simulink model "Final_V2"  (R2024a)
settings: Solver=FixedStepAuto  StartTime=0.0  StopTime=inf  FixedStep=1e-3  RelTol=1e-3
libraries: fl_lib x107, nesl_utility x75, ee_lib x16, batteryecm_lib x14, simulink x2
(part 16 of 16)

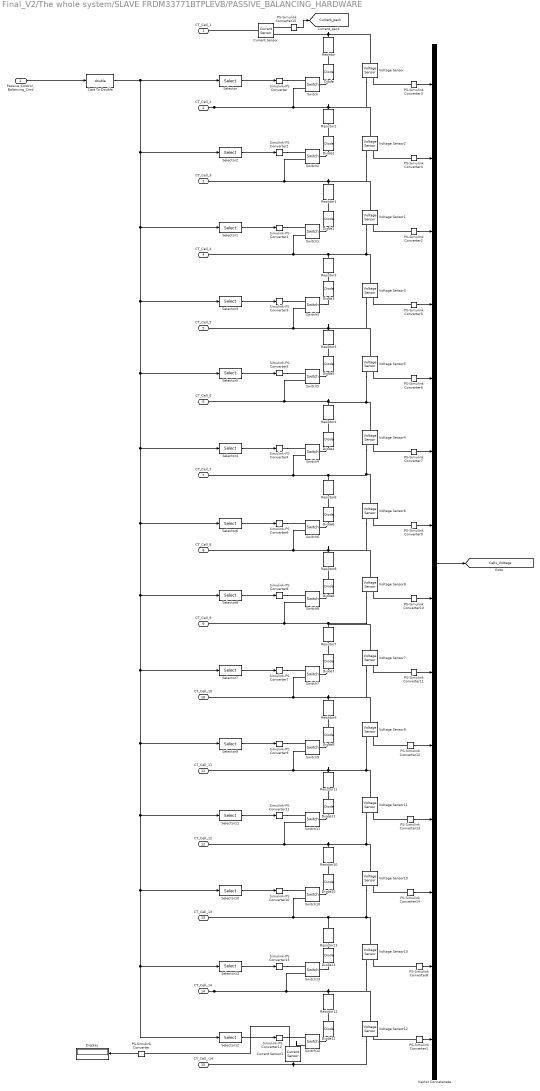
== system Final_V2/The whole system/SLAVE FRDM33771BTPLEVB/PASSIVE_BALANCING_HARDWARE ==
  Passive_Control_Balancing_Cmd : Inport
  Cast To Double : DataTypeConversion  OutDataTypeStr=double  SaturateOnIntegerOverflow=off  RndMeth=Floor
  Current Sensor : fl_lib/Electrical/Electrical Sensors/Current Sensor
  Current Sensor1 : fl_lib/Electrical/Electrical Sensors/Current Sensor
  Diode{,1..13} (14 alike) : ee_lib/Semiconductors & Converters/Diode  ModelType=ee.enum.diode.modelType.pwl  TableType=ee.enum.diode.tableType.IfMat  IfMat=[.07, .12, .19, 1.75, 4.24, 7.32, 11.2; .16, .3, .72, 2.14, 5.02, 8.35, 13.12] A  VfMat=[.9, 1.15, 1.25, 1.5, 1.75, 2.17, 2.6, 2.85; .58, .68, .75, 1.1, 1.38, 1.77, 2.27, 2.7] V  TjVec=[25, 125] degC  VfVec=[.5, .7, .9, 1.3, 1.7, 2.1, 2.5] V  IfVec=[.1, .2, .5, 1, 2, 4, 7, 10] A  IrMat=[1e-06, 2e-06, 5e-06, 1e-05, 2.5e-05, 5e-05, .0001; 2e-06, 5e-06, 1e-05, 4e-05, 8e-05, .0002, .0005] A  VrMat=[10, 100, 200, 250, 300, 325, 350; 10, 70, 160, 220, 270, 300, 320] V  VrVec=[10, 20, 40, 50, 70, 80, 100] V  IrVec=[1e-06, 2e-06, 5e-06, 1e-05, 2e-05, 5e-05, .0001] A  Vf=0.6 V  Ron=0.3 Ohm  Goff=1e-8 S  prmExp=ee.enum.diode.expParam.useTwo  I12=[.0137, .545] A  V12=[.6, .7] V  RS=0 Ohm  Tmeas=298.15 K  IS=1e-12 A  ec=1  I1=0.0137 A  V1=0.6 V  N_series=1  N_parallel=1  ZenerEnable=false  Rz=0.3 Ohm  BV=inf V  C_param=ee.enum.diode.capParam.fixed  CJ=0 pF  CJ0=5 pF  Vvec=[.1, 10, 100] V  Cvec=[3.5, 1, .4] pF  VJ=1 V  Mgrad=0.5  FC=0.5  Q_param=ee.enum.diode.recoveryParam.off  iRM=-7.15 A  iF=4 A  didt=-750 A/us  trr_factor=3  trr=115 ns  Qrr=150 nA*s  Vr=-600 V  Ls=150 nH  Erec=0.03 J  TT=50 ns  tau=100 ns  T_param=ee.enum.diode.temperatureParam.off  Tdevice=298.15 K  I1_T2=0.245 A  V1_T2=0.5 V  IS_T2=1.25e-7 A  Tmeas2=125 degC  XTI_param=ee.enum.diode.xtiParam.pn  XTI=3  EG_param=ee.enum.diode.egapParam.material_si  EG=1.11 eV  dBVdT=0 V/K  enable_faults=simscape.enum.onoff.off  fault_action=simscape.enum.assert.action.none  fault_types=ee.enum.diode.faults.open  tau_fault=1e-3 s  IfMat_faulted=[.07, .12, .19, 1.75, 4.24, 7.32, 11.2; .16, .3, .72, 2.14, 4.02, 6.35, 9.12] A  VfMat_faulted=[.9, 1.15, 1.25, 1.5, 1.75, 2.17, 2.6, 2.85; .58, .68, .75, 1.1, 1.38, 1.77, 2.27, 2.7] V  VfVec_faulted=[.5, .7, .9, 1.3, 1.7, 2.1, 2.5] V  IfVec_faulted=[.1, .2, .5, 1, 2, 4, 7, 10] A  IrMat_faulted=[1e-06, 2e-06, 5e-06, 1e-05, 2.5e-05, 5e-05, .0001; 2e-06, 5e-06, 1e-05, 4e-05, 8e-05, .0002, .0005] A  VrMat_faulted=[1, 10, 20, 25, 30, 32.5, 35; 1, 5, 10, 20, 25, 30, 32] V  VrVec_faulted=[1, 2, 4, 5, 7, 8, 10] V  IrVec_faulted=[1e-06, 2e-06, 5e-06, 1e-05, 2e-05, 5e-05, .0001] A  Ropen_faulted=1000 Ohm  Gshort_faulted=1000 1/Ohm  Vf_faulted=0.6 V  Ron_faulted=0.3 Ohm  Goff_faulted=1e-8 S  I12_faulted=[.0137, .545] A  V12_faulted=[.6, .7] V  IS_faulted=1e-12 A  ec_faulted=1  I1_faulted=0.0137 A  V1_faulted=0.6 V  RS_faulted=0 Ohm  Rz_faulted=0.3 Ohm  BV_faulted=inf V  Cscale_faulted=100  fault_trigger=ee.enum.faults.faultTrigger.temporal  t_fault=inf s  max_permissible_voltage=inf V  n_fail_on_voltage=1  max_permissible_current=inf A  t_fail_on_current=1 s  max_permissible_dVdt=inf V/s  enable_fault_input=simscape.enum.onoff.off  i_specify=off  i_priority=None  i=0 A  i_nominal_specify=off  i_nominal_value=1  i_nominal_unit=A  v_specify=off  v_priority=None  v=0 V  v_nominal_specify=off  v_nominal_value=1  v_nominal_unit=V  v_capacitor_specify=off  v_capacitor_priority=None  v_capacitor=0 V  v_capacitor_nominal_specify=off  v_capacitor_nominal_value=1  v_capacitor_nominal_unit=V  Qj_specify=off  Qj_priority=High  Qj=0 C  Qj_nominal_specify=off  Qj_nominal_value=1  Qj_nominal_unit=C  faulted_specify=off  faulted_priority=None  faulted=0  faulted_nominal_specify=off  faulted_nominal_value=1  faulted_nominal_unit=1  i_diode_specify=off  i_diode_priority=None  i_diode=0 A  i_diode_nominal_specify=off  i_diode_nominal_value=1  i_diode_nominal_unit=A  v_diode_specify=off  v_diode_priority=None  v_diode=0 V  v_diode_nominal_specify=off  v_diode_nominal_value=1  v_diode_nominal_unit=V  i_capacitor_specify=off  i_capacitor_priority=None  i_capacitor=0 A  i_capacitor_nominal_specify=off  i_capacitor_nominal_value=1  i_capacitor_nominal_unit=A  power_dissipated_specify=off  power_dissipated_priority=None  power_dissipated=0 W  power_dissipated_nominal_specify=off  power_dissipated_nominal_value=1  power_dissipated_nominal_unit=W  fidelity_level=ee.enum.diode.fidelity.includeChargeDyn  rec_loss_model=ee.enum.diode.reverseRecoveryModel.fixed  ErecVal=0 J  scaleWithIandV=true  ErecMat=zeros(2, 3) J  TjrecVec=[298.15, 398.15] K  IfrecVec=[.1, 1, 10] A  IrecVal=10 A  VrecVal=10 V  tFilter=1 / 1000 / 100 s  max_permissible_temperature=inf K  t_fail_on_temperature=1 s  thermal_network_parameterization=ee.enum.thermalNetworkTopology.junctionCase  Rth=[0, 10] K/W  Rth_Cauer=[1, 3, 10] K/W  Rth_Foster=[4, 6] K/W  thermal_mass_parameterization=ee.enum.thermalNetwork.timeconstant  thermal_mass=[0, 1] J/K  thermal_mass_Cauer=[.1, .3, 1] J/K  thermal_mass_Foster=[1.5, 3] J/K  thermal_time_constant=[0, 10] s  thermal_time_constant_Cauer=[1, 3, 10] s  thermal_time_constant_Foster=[6, 18] s  thermal_mass_initial_temperatures=[25, 25] degC  thermal_mass_initial_temperatures_Cauer=[25, 25, 25] degC  junction_thermal_mass=0.01 J/K  Tvec_initial_Foster=[25, 25, 25, 25] degC  no_thermal_network=0  junction_temperature_specify=off  junction_temperature_priority=None  junction_temperature=25 degC  junction_temperature_nominal_specify=off  junction_temperature_nominal_value=1  junction_temperature_nominal_unit=degC
  Display : Display
  PS-Simulink Converter : nesl_utility/PS-Simulink Converter  PhysicalDomain=network_engine_domain  SubClassName=ps_output  LeftPortType=input  RightPortType=output  PseudoPeriodic=off  Frequencies=[]  VectorFormat=inherit  Unit=inherit  AffineConversion=off
  PS-Simulink Converter{1..15} (15 alike) : nesl_utility/PS-Simulink Converter  PhysicalDomain=network_engine_domain  SubClassName=ps_output  LeftPortType=input  RightPortType=output  PseudoPeriodic=off  Frequencies=[]  VectorFormat=1-D array  Unit=inherit  AffineConversion=off
  Resistor{,1..13} (14 alike) : fl_lib/Electrical/Electrical Elements/Resistor  R=1 Ohm  i_specify=off  i_priority=None  i=0 A  i_nominal_specify=off  i_nominal_value=1  i_nominal_unit=A  v_specify=off  v_priority=None  v=0 V  v_nominal_specify=off  v_nominal_value=1  v_nominal_unit=V
  Selector : Selector  InputPortWidth=14  IndexOptions=Index vector (dialog)  Indices=1  OutputSizes=1
  Selector1 : Selector  InputPortWidth=14  IndexOptions=Index vector (dialog)  Indices=3  OutputSizes=1
  Selector10 : Selector  InputPortWidth=14  IndexOptions=Index vector (dialog)  Indices=12  OutputSizes=1
  Selector11 : Selector  InputPortWidth=14  IndexOptions=Index vector (dialog)  Indices=11  OutputSizes=1
  Selector12 : Selector  InputPortWidth=14  IndexOptions=Index vector (dialog)  Indices=14  OutputSizes=1
  Selector13 : Selector  InputPortWidth=14  IndexOptions=Index vector (dialog)  Indices=13  OutputSizes=1
  Selector2 : Selector  InputPortWidth=14  IndexOptions=Index vector (dialog)  Indices=2  OutputSizes=1
  Selector3 : Selector  InputPortWidth=14  IndexOptions=Index vector (dialog)  Indices=4  OutputSizes=1
  Selector4 : Selector  InputPortWidth=14  IndexOptions=Index vector (dialog)  Indices=6  OutputSizes=1
  Selector5 : Selector  InputPortWidth=14  IndexOptions=Index vector (dialog)  Indices=5  OutputSizes=1
  Selector6 : Selector  InputPortWidth=14  IndexOptions=Index vector (dialog)  Indices=7  OutputSizes=1
  Selector7 : Selector  InputPortWidth=14  IndexOptions=Index vector (dialog)  Indices=9  OutputSizes=1
  Selector8 : Selector  InputPortWidth=14  IndexOptions=Index vector (dialog)  Indices=8  OutputSizes=1
  Selector9 : Selector  InputPortWidth=14  IndexOptions=Index vector (dialog)  Indices=10  OutputSizes=1
  Simulink-PS Converter{,1..13} (14 alike) : nesl_utility/Simulink-PS Converter  PhysicalDomain=network_engine_domain  SubClassName=ps_input  LeftPortType=input  RightPortType=output  PseudoPeriodic=off  Frequencies=[]  NoiseDistribution=none  NoiseParameters=[]  Unit=1  AffineConversion=off  FilteringAndDerivatives=provide  SimscapeFilterOrder=1  InputFilterTimeConstant=0.001  UdotUserProvided=0
  Switch{,1..13} (14 alike) : fl_lib/Electrical/Electrical Elements/Switch  R_closed=0.01 Ohm  G_open=1e-8 1/Ohm  Threshold=0.5  i_specify=off  i_priority=None  i=0 A  i_nominal_specify=off  i_nominal_value=1  i_nominal_unit=A  v_specify=off  v_priority=None  v=0 V  v_nominal_specify=off  v_nominal_value=1  v_nominal_unit=V
  Vector Concatenate : Concatenate  NumInputs=14
  Voltage Sensor{,1..13} (14 alike) : fl_lib/Electrical/Electrical Sensors/Voltage Sensor
  CT_Cell_1 : PMIOPort  Side=Left
  CT_Cell_2 : PMIOPort  Port=2  Side=Left
  CT_Cell_3 : PMIOPort  Port=3  Side=Left
  CT_Cell_4 : PMIOPort  Port=4  Side=Left
  CT_Cell_5 : PMIOPort  Port=5  Side=Left
  CT_Cell_6 : PMIOPort  Port=6  Side=Left
  CT_Cell_7 : PMIOPort  Port=7  Side=Left
  CT_Cell_8 : PMIOPort  Port=8  Side=Left
  CT_Cell_9 : PMIOPort  Port=9  Side=Left
  CT_Cell_10 : PMIOPort  Port=10  Side=Left
  CT_Cell_11 : PMIOPort  Port=11  Side=Left
  CT_Cell_12 : PMIOPort  Port=12  Side=Left
  CT_Cell_13 : PMIOPort  Port=13  Side=Left
  CT_Cell_14 : PMIOPort  Port=14  Side=Left
  CT_Cell_-14 : PMIOPort  Port=15  Side=Left
  tag scope: Cells_Voltage=global, Current_pack=global
  wires:
  PS-Simulink Converter11/1 -> Vector Concatenate/9
  PS-Simulink Converter1/1 -> Vector Concatenate/14
  PS-Simulink Converter3/1 -> Vector Concatenate/1
  PS-Simulink Converter14/1 -> Vector Concatenate/12
  PS-Simulink Converter5/1 -> Vector Concatenate/4
  Selector9/1 -> Simulink-PS Converter9/1
  Selector6/1 -> Simulink-PS Converter6/1
  Selector7/1 -> Simulink-PS Converter7/1
  Selector12/1 -> Simulink-PS Converter12/1
  Selector13/1 -> Simulink-PS Converter13/1
  PS-Simulink Converter7/1 -> Vector Concatenate/6
  Selector1/1 -> Simulink-PS Converter1/1
  PS-Simulink Converter9/1 -> Vector Concatenate/7
  PS-Simulink Converter2/1 -> Vector Concatenate/3
  Selector5/1 -> Simulink-PS Converter5/1
  PS-Simulink Converter4/1 -> Vector Concatenate/2
  Selector2/1 -> Simulink-PS Converter2/1
  PS-Simulink Converter10/1 -> Vector Concatenate/8
  Vector Concatenate/1 -> [Cells_Voltage]
  PS-Simulink Converter8/1 -> Vector Concatenate/13
  Selector/1 -> Simulink-PS Converter/1
  PS-Simulink Converter12/1 -> Vector Concatenate/10
  Selector10/1 -> Simulink-PS Converter10/1
  Selector8/1 -> Simulink-PS Converter8/1
  Selector3/1 -> Simulink-PS Converter3/1
  Selector11/1 -> Simulink-PS Converter11/1
  PS-Simulink Converter6/1 -> Vector Concatenate/5
  Selector4/1 -> Simulink-PS Converter4/1
  PS-Simulink Converter13/1 -> Vector Concatenate/11
  Cast To Double/1 -> Selector2/1, Selector1/1, Selector3/1, Selector5/1, Selector4/1, Selector6/1, Selector8/1, Selector9/1, Selector11/1, Selector10/1, Selector13/1, Selector12/1, Selector7/1, Selector/1
  Passive_Control_Balancing_Cmd/1 -> Cast To Double/1
  PS-Simulink Converter15/1 -> [Current_pack]
  PS-Simulink Converter/1 -> Display/1
  connections:
  Voltage Sensor9/RConn2 -- Voltage Sensor11/LConn1 -- Resistor11/LConn1 -- Switch9/RConn2 -- CT_Cell_11/RConn1
  CT_Cell_14/RConn1 -- Switch13/RConn2 -- Voltage Sensor12/LConn1 -- Voltage Sensor13/RConn2 -- Resistor12/LConn1
  Voltage Sensor4/RConn2 -- Voltage Sensor6/LConn1 -- Resistor6/LConn1 -- Switch4/RConn2 -- CT_Cell_7/RConn1
  Resistor1/LConn1 -- Voltage Sensor1/LConn1 -- Voltage Sensor2/RConn2 -- Switch2/RConn2 -- CT_Cell_3/RConn1
  Voltage Sensor4/LConn1 -- Voltage Sensor5/RConn2 -- Resistor4/LConn1 -- Switch5/RConn2 -- CT_Cell_6/RConn1
  Resistor8/LConn1 -- Voltage Sensor8/LConn1 -- Voltage Sensor6/RConn2 -- Switch6/RConn2 -- CT_Cell_8/RConn1
  Switch3/RConn2 -- Voltage Sensor5/LConn1 -- Voltage Sensor3/RConn2 -- Resistor5/LConn1 -- CT_Cell_5/RConn1
  Voltage Sensor11/RConn2 -- Voltage Sensor10/LConn1 -- Resistor10/LConn1 -- Switch11/RConn2 -- CT_Cell_12/RConn1
  Resistor7/LConn1 -- Voltage Sensor8/RConn2 -- Voltage Sensor7/LConn1 -- Switch8/RConn2 -- CT_Cell_9/RConn1
  Voltage Sensor10/RConn2 -- Voltage Sensor13/LConn1 -- Resistor13/LConn1 -- Switch10/RConn2 -- CT_Cell_13/RConn1
  Voltage Sensor12/RConn2 -- Current Sensor1/LConn1 -- CT_Cell_-14/RConn1
  Voltage Sensor1/RConn2 -- Voltage Sensor3/LConn1 -- Resistor3/LConn1 -- Switch1/RConn2 -- CT_Cell_4/RConn1
  Resistor/LConn1 -- Voltage Sensor/LConn1 -- Current Sensor/RConn2
  Resistor9/LConn1 -- Voltage Sensor9/LConn1 -- Voltage Sensor7/RConn2 -- Switch7/RConn2 -- CT_Cell_10/RConn1
  CT_Cell_2/RConn1 -- Voltage Sensor2/LConn1 -- Voltage Sensor/RConn2 -- Resistor2/LConn1 -- Switch/RConn2
  PS-Simulink Converter13/LConn1 -- Voltage Sensor11/RConn1
  Simulink-PS Converter13/RConn1 -- Switch13/RConn1
  Simulink-PS Converter5/RConn1 -- Switch5/RConn1
  PS-Simulink Converter3/LConn1 -- Voltage Sensor/RConn1
  Diode6/LConn1 -- Resistor6/RConn1
  Switch6/LConn1 -- Diode6/RConn1
  PS-Simulink Converter6/LConn1 -- Voltage Sensor5/RConn1
  Diode7/LConn1 -- Resistor7/RConn1
  Diode2/LConn1 -- Resistor2/RConn1
  Diode1/LConn1 -- Resistor1/RConn1
  Switch9/LConn1 -- Diode9/RConn1
  Simulink-PS Converter10/RConn1 -- Switch10/RConn1
  Diode11/LConn1 -- Resistor11/RConn1
  Resistor13/RConn1 -- Diode13/LConn1
  PS-Simulink Converter4/LConn1 -- Voltage Sensor2/RConn1
  Switch10/LConn1 -- Diode10/RConn1
  Switch13/LConn1 -- Diode13/RConn1
  Simulink-PS Converter11/RConn1 -- Switch11/RConn1
  PS-Simulink Converter8/LConn1 -- Voltage Sensor13/RConn1
  Diode9/LConn1 -- Resistor9/RConn1
  Diode3/LConn1 -- Resistor3/RConn1
  Switch3/LConn1 -- Diode3/RConn1
  Diode12/LConn1 -- Resistor12/RConn1
  Simulink-PS Converter2/RConn1 -- Switch2/RConn1
  Simulink-PS Converter6/RConn1 -- Switch6/RConn1
  Simulink-PS Converter1/RConn1 -- Switch1/RConn1
  Switch2/LConn1 -- Diode2/RConn1
  PS-Simulink Converter2/LConn1 -- Voltage Sensor1/RConn1
  Switch5/LConn1 -- Diode5/RConn1
  Switch7/LConn1 -- Diode7/RConn1
  Diode5/LConn1 -- Resistor5/RConn1
  Simulink-PS Converter8/RConn1 -- Switch8/RConn1
  PS-Simulink Converter11/LConn1 -- Voltage Sensor7/RConn1
  Switch/LConn1 -- Diode/RConn1
  Simulink-PS Converter/RConn1 -- Switch/RConn1
  Simulink-PS Converter9/RConn1 -- Switch9/RConn1
  PS-Simulink Converter5/LConn1 -- Voltage Sensor3/RConn1
  PS-Simulink Converter7/LConn1 -- Voltage Sensor4/RConn1
  Diode8/LConn1 -- Resistor8/RConn1
  PS-Simulink Converter10/LConn1 -- Voltage Sensor8/RConn1
  PS-Simulink Converter9/LConn1 -- Voltage Sensor6/RConn1
  Simulink-PS Converter12/RConn1 -- Switch12/RConn1
  PS-Simulink Converter14/LConn1 -- Voltage Sensor10/RConn1
  Switch11/LConn1 -- Diode11/RConn1
  Diode10/LConn1 -- Resistor10/RConn1
  Simulink-PS Converter3/RConn1 -- Switch3/RConn1
  PS-Simulink Converter12/LConn1 -- Voltage Sensor9/RConn1
  Diode4/LConn1 -- Resistor4/RConn1
  Diode/LConn1 -- Resistor/RConn1
  Switch1/LConn1 -- Diode1/RConn1
  Switch12/LConn1 -- Diode12/RConn1
  PS-Simulink Converter1/LConn1 -- Voltage Sensor12/RConn1
  Simulink-PS Converter7/RConn1 -- Switch7/RConn1
  Simulink-PS Converter4/RConn1 -- Switch4/RConn1
  Switch8/LConn1 -- Diode8/RConn1
  Switch4/LConn1 -- Diode4/RConn1
  Current Sensor/LConn1 -- CT_Cell_1/RConn1
  PS-Simulink Converter15/LConn1 -- Current Sensor/RConn1
  Current Sensor1/RConn2 -- Switch12/RConn2
  Current Sensor1/RConn1 -- PS-Simulink Converter/LConn1

== system Final_V2/The whole system/SLAVE FRDM33771BTPLEVB/Tempreture_Of_Cells ==
  Temp.13&14 : Inport
  Temp.11&12 : Inport  Port=2
  Temp.9&10 : Inport  Port=3
  Temp.7&8 : Inport  Port=4
  Temp.5&6 : Inport  Port=5
  Temp.3&4 : Inport  Port=6
  Temp.1&2 : Inport  Port=7
  Bus Creator : BusCreator  Inputs=7
  tag scope: Temp_Sensing=global
  wires:
  Temp.1&2/1 -> Bus Creator/1  "Temp_1&2"
  Temp.3&4/1 -> Bus Creator/2  "Temp_3&4"
  Temp.5&6/1 -> Bus Creator/3  "Temp_5&6"
  Temp.7&8/1 -> Bus Creator/4  "Temp_7&8"
  Temp.9&10/1 -> Bus Creator/5  "Temp_9&10"
  Temp.11&12/1 -> Bus Creator/6  "Temp_11&12"
  Temp.13&14/1 -> Bus Creator/7  "Temp_13&14"
  Bus Creator/1 -> [Temp_Sensing]

== system Final_V2/The whole system/Master/Subsystem15/Subsystem1 ==
  soc_intial_cell_2 : Inport
  Current_Branch : Inport  Port=2
  "\"Ah\"" : Gain  Gain=1/1.5
  Constant : Constant  Value=0
  Integrator : Integrator
  Sum : Sum
  Switch : Switch  Criteria=u2 > Threshold  SaturateOnIntegerOverflow=off  InputSameDT=off
  To convert Ah -->Coulomb : Gain  Gain=1/3600
  SOC_CELL_2 : Outport
  wires:
  soc_intial_cell_2/1 -> Sum/1
  Current_Branch/1 -> Integrator/1
  Integrator/1 -> "\"Ah\""/1  "Integrated current"
  Constant/1 -> Switch/3
  To convert Ah -->Coulomb/1 -> Sum/2  "Integrated current / Coulomb"
  Switch/1 -> SOC_CELL_2/1
  "\"Ah\""/1 -> To convert Ah -->Coulomb/1
  Sum/1 -> Switch/1, Switch/2

== system Final_V2/The whole system/Master/Subsystem15/Subsystem10 ==
  soc_intial_cell_11 : Inport
  Current_Branch : Inport  Port=2
  "\"Ah\"" : Gain  Gain=1/1.5
  Constant : Constant  Value=0
  Integrator : Integrator
  Sum : Sum
  Switch : Switch  Criteria=u2 > Threshold  SaturateOnIntegerOverflow=off  InputSameDT=off
  To convert Ah -->Coulomb : Gain  Gain=1/3600
  SOC_CELL_11 : Outport
  wires:
  Sum/1 -> Switch/2, Switch/1
  "\"Ah\""/1 -> To convert Ah -->Coulomb/1
  Switch/1 -> SOC_CELL_11/1
  To convert Ah -->Coulomb/1 -> Sum/2  "Integrated current / Coulomb"
  Constant/1 -> Switch/3
  Integrator/1 -> "\"Ah\""/1  "Integrated current"
  Current_Branch/1 -> Integrator/1
  soc_intial_cell_11/1 -> Sum/1

== system Final_V2/The whole system/Master/Subsystem15/Subsystem11 ==
  soc_intial_cell_12 : Inport
  Current_Branch : Inport  Port=2
  "\"Ah\"" : Gain  Gain=1/1.5
  Constant : Constant  Value=0
  Integrator : Integrator
  Sum : Sum
  Switch : Switch  Criteria=u2 > Threshold  SaturateOnIntegerOverflow=off  InputSameDT=off
  To convert Ah -->Coulomb : Gain  Gain=1/3600
  SOC_CELL_12 : Outport
  wires:
  soc_intial_cell_12/1 -> Sum/1
  Current_Branch/1 -> Integrator/1
  Integrator/1 -> "\"Ah\""/1  "Integrated current"
  Constant/1 -> Switch/3
  To convert Ah -->Coulomb/1 -> Sum/2  "Integrated current / Coulomb"
  Switch/1 -> SOC_CELL_12/1
  "\"Ah\""/1 -> To convert Ah -->Coulomb/1
  Sum/1 -> Switch/1, Switch/2

== system Final_V2/The whole system/Master/Subsystem15/Subsystem12 ==
  soc_intial_cell_13 : Inport
  Current_Branch : Inport  Port=2
  "\"Ah\"" : Gain  Gain=1/1.5
  Constant : Constant  Value=0
  Integrator : Integrator
  Sum : Sum
  Switch : Switch  Criteria=u2 > Threshold  SaturateOnIntegerOverflow=off  InputSameDT=off
  To convert Ah -->Coulomb : Gain  Gain=1/3600
  SOC_CELL_13 : Outport
  wires:
  Sum/1 -> Switch/2, Switch/1
  "\"Ah\""/1 -> To convert Ah -->Coulomb/1
  Switch/1 -> SOC_CELL_13/1
  To convert Ah -->Coulomb/1 -> Sum/2  "Integrated current / Coulomb"
  Constant/1 -> Switch/3
  Integrator/1 -> "\"Ah\""/1  "Integrated current"
  Current_Branch/1 -> Integrator/1
  soc_intial_cell_13/1 -> Sum/1

== system Final_V2/The whole system/Master/Subsystem15/Subsystem13 ==
  soc_intial_cell_12 : Inport
  Current_Branch : Inport  Port=2
  "\"Ah\"" : Gain  Gain=1/1.5
  Constant : Constant  Value=0
  Integrator : Integrator
  Sum : Sum
  Switch : Switch  Criteria=u2 > Threshold  SaturateOnIntegerOverflow=off  InputSameDT=off
  To convert Ah -->Coulomb : Gain  Gain=1/3600
  SOC_CELL_14 : Outport
  wires:
  soc_intial_cell_12/1 -> Sum/1
  Current_Branch/1 -> Integrator/1
  Integrator/1 -> "\"Ah\""/1  "Integrated current"
  Constant/1 -> Switch/3
  To convert Ah -->Coulomb/1 -> Sum/2  "Integrated current / Coulomb"
  Switch/1 -> SOC_CELL_14/1
  "\"Ah\""/1 -> To convert Ah -->Coulomb/1
  Sum/1 -> Switch/1, Switch/2

== system Final_V2/The whole system/Master/Subsystem15/Subsystem14 ==
  soc_intial_cell_1 : Inport
  Current_Branch : Inport  Port=2
  "\"Ah\"" : Gain  Gain=1/1.5
  Constant : Constant  Value=0
  Integrator : Integrator
  Sum : Sum
  Switch : Switch  Criteria=u2 > Threshold  SaturateOnIntegerOverflow=off  InputSameDT=off
  To convert Ah -->Coulomb : Gain  Gain=1/3600
  SOC_CELL_1 : Outport
  wires:
  Sum/1 -> Switch/2, Switch/1
  "\"Ah\""/1 -> To convert Ah -->Coulomb/1
  Switch/1 -> SOC_CELL_1/1
  To convert Ah -->Coulomb/1 -> Sum/2  "Integrated current / Coulomb"
  Constant/1 -> Switch/3
  Integrator/1 -> "\"Ah\""/1  "Integrated current"
  Current_Branch/1 -> Integrator/1
  soc_intial_cell_1/1 -> Sum/1

== system Final_V2/The whole system/Master/Subsystem15/Subsystem2 ==
  soc_intial_cell_3 : Inport
  Current_Branch : Inport  Port=2
  "\"Ah\"" : Gain  Gain=1/1.5
  Constant : Constant  Value=0
  Integrator : Integrator
  Sum : Sum
  Switch : Switch  Criteria=u2 > Threshold  SaturateOnIntegerOverflow=off  InputSameDT=off
  To convert Ah -->Coulomb : Gain  Gain=1/3600
  SOC_CELL_3 : Outport
  wires:
  Sum/1 -> Switch/2, Switch/1
  "\"Ah\""/1 -> To convert Ah -->Coulomb/1
  Switch/1 -> SOC_CELL_3/1
  To convert Ah -->Coulomb/1 -> Sum/2  "Integrated current / Coulomb"
  Constant/1 -> Switch/3
  Integrator/1 -> "\"Ah\""/1  "Integrated current"
  Current_Branch/1 -> Integrator/1
  soc_intial_cell_3/1 -> Sum/1

== system Final_V2/The whole system/Master/Subsystem15/Subsystem3 ==
  soc_intial_cell_4 : Inport
  Current_Branch : Inport  Port=2
  "\"Ah\"" : Gain  Gain=1/1.5
  Constant : Constant  Value=0
  Integrator : Integrator
  Sum : Sum
  Switch : Switch  Criteria=u2 > Threshold  SaturateOnIntegerOverflow=off  InputSameDT=off
  To convert Ah -->Coulomb : Gain  Gain=1/3600
  SOC_CELL_4 : Outport
  wires:
  soc_intial_cell_4/1 -> Sum/1
  Current_Branch/1 -> Integrator/1
  Integrator/1 -> "\"Ah\""/1  "Integrated current"
  Constant/1 -> Switch/3
  To convert Ah -->Coulomb/1 -> Sum/2  "Integrated current / Coulomb"
  Switch/1 -> SOC_CELL_4/1
  "\"Ah\""/1 -> To convert Ah -->Coulomb/1
  Sum/1 -> Switch/1, Switch/2

== system Final_V2/The whole system/Master/Subsystem15/Subsystem4 ==
  soc_intial_cell_5 : Inport
  Current_Branch : Inport  Port=2
  "\"Ah\"" : Gain  Gain=1/1.5
  Constant : Constant  Value=0
  Integrator : Integrator
  Sum : Sum
  Switch : Switch  Criteria=u2 > Threshold  SaturateOnIntegerOverflow=off  InputSameDT=off
  To convert Ah -->Coulomb : Gain  Gain=1/3600
  SOC_CELL_5 : Outport
  wires:
  Sum/1 -> Switch/2, Switch/1
  "\"Ah\""/1 -> To convert Ah -->Coulomb/1
  Switch/1 -> SOC_CELL_5/1
  To convert Ah -->Coulomb/1 -> Sum/2  "Integrated current / Coulomb"
  Constant/1 -> Switch/3
  Integrator/1 -> "\"Ah\""/1  "Integrated current"
  Current_Branch/1 -> Integrator/1
  soc_intial_cell_5/1 -> Sum/1

== system Final_V2/The whole system/Master/Subsystem15/Subsystem5 ==
  soc_intial_cell_6 : Inport
  Current_Branch : Inport  Port=2
  "\"Ah\"" : Gain  Gain=1/1.5
  Constant : Constant  Value=0
  Integrator : Integrator
  Sum : Sum
  Switch : Switch  Criteria=u2 > Threshold  SaturateOnIntegerOverflow=off  InputSameDT=off
  To convert Ah -->Coulomb : Gain  Gain=1/3600
  SOC_CELL_6 : Outport
  wires:
  soc_intial_cell_6/1 -> Sum/1
  Current_Branch/1 -> Integrator/1
  Integrator/1 -> "\"Ah\""/1  "Integrated current"
  Constant/1 -> Switch/3
  To convert Ah -->Coulomb/1 -> Sum/2  "Integrated current / Coulomb"
  Switch/1 -> SOC_CELL_6/1
  "\"Ah\""/1 -> To convert Ah -->Coulomb/1
  Sum/1 -> Switch/1, Switch/2

== system Final_V2/The whole system/Master/Subsystem15/Subsystem6 ==
  soc_intial_cell_7 : Inport
  Current_Branch : Inport  Port=2
  "\"Ah\"" : Gain  Gain=1/1.5
  Constant : Constant  Value=0
  Integrator : Integrator
  Sum : Sum
  Switch : Switch  Criteria=u2 > Threshold  SaturateOnIntegerOverflow=off  InputSameDT=off
  To convert Ah -->Coulomb : Gain  Gain=1/3600
  SOC_CELL_7 : Outport
  wires:
  Sum/1 -> Switch/2, Switch/1
  "\"Ah\""/1 -> To convert Ah -->Coulomb/1
  Switch/1 -> SOC_CELL_7/1
  To convert Ah -->Coulomb/1 -> Sum/2  "Integrated current / Coulomb"
  Constant/1 -> Switch/3
  Integrator/1 -> "\"Ah\""/1  "Integrated current"
  Current_Branch/1 -> Integrator/1
  soc_intial_cell_7/1 -> Sum/1

== system Final_V2/The whole system/Master/Subsystem15/Subsystem7 ==
  soc_intial_cell_8 : Inport
  Current_Branch : Inport  Port=2
  "\"Ah\"" : Gain  Gain=1/1.5
  Constant : Constant  Value=0
  Integrator : Integrator
  Sum : Sum
  Switch : Switch  Criteria=u2 > Threshold  SaturateOnIntegerOverflow=off  InputSameDT=off
  To convert Ah -->Coulomb : Gain  Gain=1/3600
  SOC_CELL_8 : Outport
  wires:
  soc_intial_cell_8/1 -> Sum/1
  Current_Branch/1 -> Integrator/1
  Integrator/1 -> "\"Ah\""/1  "Integrated current"
  Constant/1 -> Switch/3
  To convert Ah -->Coulomb/1 -> Sum/2  "Integrated current / Coulomb"
  Switch/1 -> SOC_CELL_8/1
  "\"Ah\""/1 -> To convert Ah -->Coulomb/1
  Sum/1 -> Switch/1, Switch/2

== system Final_V2/The whole system/Master/Subsystem15/Subsystem8 ==
  soc_intial_cell_9 : Inport
  Current_Branch : Inport  Port=2
  "\"Ah\"" : Gain  Gain=1/1.5
  Constant : Constant  Value=0
  Integrator : Integrator
  Sum : Sum
  Switch : Switch  Criteria=u2 > Threshold  SaturateOnIntegerOverflow=off  InputSameDT=off
  To convert Ah -->Coulomb : Gain  Gain=1/3600
  SOC_CELL_9 : Outport
  wires:
  Sum/1 -> Switch/2, Switch/1
  "\"Ah\""/1 -> To convert Ah -->Coulomb/1
  Switch/1 -> SOC_CELL_9/1
  To convert Ah -->Coulomb/1 -> Sum/2  "Integrated current / Coulomb"
  Constant/1 -> Switch/3
  Integrator/1 -> "\"Ah\""/1  "Integrated current"
  Current_Branch/1 -> Integrator/1
  soc_intial_cell_9/1 -> Sum/1

== system Final_V2/The whole system/Master/Subsystem15/Subsystem9 ==
  soc_intial_cell_10 : Inport
  Current_Branch : Inport  Port=2
  "\"Ah\"" : Gain  Gain=1/1.5
  Constant : Constant  Value=0
  Integrator : Integrator
  Sum : Sum
  Switch : Switch  Criteria=u2 > Threshold  SaturateOnIntegerOverflow=off  InputSameDT=off
  To convert Ah -->Coulomb : Gain  Gain=1/3600
  SOC_CELL_10 : Outport
  wires:
  soc_intial_cell_10/1 -> Sum/1
  Current_Branch/1 -> Integrator/1
  Integrator/1 -> "\"Ah\""/1  "Integrated current"
  Constant/1 -> Switch/3
  To convert Ah -->Coulomb/1 -> Sum/2  "Integrated current / Coulomb"
  Switch/1 -> SOC_CELL_10/1
  "\"Ah\""/1 -> To convert Ah -->Coulomb/1
  Sum/1 -> Switch/1, Switch/2

== system Final_V2/The whole system/SLAVE FRDM33771BTPLEVB/Fault_detection/temp fault ==
  note: Tempreture Faults
  note: Voltages Faults
  Display : Display
  Display1 : Display
  MATLAB Function : MATLAB Function  TreatAsAtomicUnit=on
  MATLAB Function1 : MATLAB Function  TreatAsAtomicUnit=on
  OR : Logic  Operator=OR  IconShape=distinctive  AllPortsSameDT=off  OutDataTypeStr=boolean
  Terminator{,1..3} (4 alike) : Terminator
  Fault_Flag : Outport
  wires:
  MATLAB Function/1(battery_index) -> Terminator/1
  [Temp_Sensing] -> MATLAB Function/1(temps)
  MATLAB Function1/4(Switchs) -> Terminator3/1
  MATLAB Function1/2(Under_voltage) -> Terminator2/1
  [Cells_Voltage] -> MATLAB Function1/1(voltages)
  MATLAB Function1/1(Over_voltage) -> Terminator1/1
  MATLAB Function1/3(Volt_Flag) -> Display/1, OR/1  "Volt_Flag"
  MATLAB Function/2(Temp_Flag) -> Display1/1, OR/2  "Temp_Flag"
  OR/1 -> Fault_Flag/1  "Fault_Flag"

== stateflow chart Final_V2/The whole system/Master/Subsystem/Chart ==
  state Enable_Pre_Charger [commented out]
    entry:
    Enable_Charger=1;
    Pre_Charger_Contactor=1;
    Negative_Charger_Contactor=1;
  state Enable_Charger [commented out]
    entry:
    Pre_Charger_Contactor=0;
    Chrger_Contactor=1;
  state Charging [commented out]
    entry:
    Enable_Charger=1;
  note: We need to add timing condition
  state init [commented out]
    entry:
    Pre_Charger_Contactor=0;
    Chrger_Contactor=0;
    Inverter_Contactor=0;
    Pre_Inverter_Contactor=0;
    Enable_Charger=0;
    Enable_Discharger=0;
    V_Battery_Min=10;
    threshold_charging=1.1;
    threshold_Discharging=38.5;
    allowable_range=2.6;
    allowable_Dis_range=5;
  state Enable_Pre_Inverter [commented out]
    entry:
    Enable_Discharger=1;
    Pre_Inverter_Contactor=1;
    Negative_Inverter_Contactor=1;
  state Enable_Inverter [commented out]
    Pre_Inverter_Contactor=0;
    Inverter_Contactor=1;
  state Discharging [commented out]
    entry:
    Enable_Discharger=1;
  note: We need to add timing condition
  state Enable_Pre_Charger1
    entry:
    Enable_Charger=1;
    Pre_Charger_Contactor=1;
    Negative_Charger_Contactor=1;
  state Enable_Charger1
    entry:
    Chrger_Contactor=1;
    Pre_Charger_Contactor=0;
  state init1
    entry:
    Pre_Charger_Contactor=0;
    Chrger_Contactor=0;
    Inverter_Contactor=0;
    Pre_Inverter_Contactor=0;
    Enable_Charger=0;
    Enable_Discharger=0;
    V_Battery_Min=10;
    threshold_charging=1.1;
    threshold_Discharging=38.5;
    allowable_range=2.6;
    allowable_Dis_range=5;
  state Enable_Inverter1
    entry:
    Inverter_Contactor=1;
    Pre_Inverter_Contactor=0;
  state Enable_Pre_Inverter1
    entry:
    Enable_Discharger=1;
    Pre_Inverter_Contactor=1;
    Negative_Inverter_Contactor=1;
  Enable_Pre_Charger -> Enable_Pre_Charger [commented out] : [Charger_Volt<(threshold_charging*Pack_Volt)]
  Enable_Charger -> Enable_Charger [commented out] : [System_State==2]
  Enable_Charger -> Charging [commented out] : after(5,sec)
  Enable_Pre_Charger -> Enable_Charger [commented out] : [(Charger_Volt>(threshold_charging*Pack_Volt))&&(Charger_Current<allowable_range)]
  init -> Enable_Pre_Charger [commented out] : [(Pack_Volt<V_Battery_Min)&&(System_State==2)]
  Enable_Pre_Charger -> init [commented out] : [System_State~=2]
  Enable_Charger -> init [commented out] : [Charger_Volt<(threshold_Discharging*Pack_Volt)]
  (default) -> init [commented out]
  Enable_Inverter -> init [commented out] : [Inverter_Volt<(threshold_charging*Pack_Volt)]
  init -> Enable_Pre_Inverter [commented out] : [(Pack_Volt>V_Battery_Min)&&(System_State==1)]
  Enable_Pre_Inverter -> init [commented out] : [System_State~=1]
  Enable_Inverter -> Discharging [commented out] : after(5,sec)
  Enable_Pre_Inverter -> Enable_Inverter [commented out] : [(Inverter_Volt>(threshold_Discharging*Pack_Volt))&&(Inverter_Current<allowable_Dis_range)]
  Enable_Pre_Inverter -> Enable_Pre_Inverter [commented out] : [Inverter_Volt<(threshold_Discharging*Pack_Volt)]
  Enable_Inverter -> Enable_Inverter [commented out] : [System_State==1]
  Enable_Charger1 -> Enable_Charger1 [order 1] : [System_State==2]
  init1 -> Enable_Pre_Charger1 [order 1] : [(System_State==2)&&(Fetal_Fault==0)&& (pack_switch==1)]
  Enable_Pre_Charger1 -> Enable_Charger1 [order 1] : after(0.4,sec)
  Enable_Pre_Charger1 -> init1 [order 2] : [System_State~=2]
  Enable_Charger1 -> init1 [order 2] : [System_State~=2]
  (default) -> init1
  Enable_Inverter1 -> init1 [order 1] : [System_State~=1]
  init1 -> Enable_Pre_Inverter1 [order 2] : [(System_State==1)&&(Fetal_Fault==0)]
  Enable_Pre_Inverter1 -> init1 [order 2] : [System_State~=1]
  Enable_Pre_Inverter1 -> Enable_Inverter1 [order 1] : after(0.4,sec)
  Enable_Inverter1 -> Enable_Inverter1 [order 2] : [System_State==1]
  data: System_State input; Charger_Volt input; Inverter_Volt input; Pack_Volt input; Chrger_Contactor output; Inverter_Contactor output; Negative_Charger_Contactor output; Negative_Inverter_Contactor output; Pre_Charger_Contactor output; Pre_Inverter_Contactor output; Enable_Charger output; Enable_Discharger output; threshold_Discharging local double size=[1 1]; threshold_charging local double size=[1 1]; allowable_range local double size=[1 1]; V_Battery_Min local double size=[1 1]; Inverter_Current input; Charger_Current input; allowable_Dis_range local; Fetal_Fault input; pack_switch input

== matlab function Final_V2/The whole system/SLAVE FRDM33771BTPLEVB/Fault_detection/temp fault/MATLAB Function ==
  data: temps input; battery_index output; Temp_Flag output
function [battery_index,Temp_Flag] = check_battery_temperature(temps)
    Temp_Flag=0;

    threshold = 353;   
    battery_index = ones(1,14);
    for i = 1:7
        if temps(i) >= threshold
            battery_index(i)=0; 
            Temp_Flag=1;
        end
    end
    
end

== matlab function Final_V2/The whole system/SLAVE FRDM33771BTPLEVB/Fault_detection/temp fault/MATLAB Function1 ==
  data: voltages input; Over_voltage output; Under_voltage output; Volt_Flag output; Switchs output
function [Over_voltage, Under_voltage, Volt_Flag, Switchs] =Voltage_Faults(voltages)
    max_threshold = 4.25;  
    min_threshold = 2.5;   
    Over_voltage= ones(1,14);
    Under_voltage= ones(1,14);
    Switchs= ones(1,14);
    Volt_Flag = 0;
    for i = 1:14
        if voltages(i) > max_threshold
            Over_voltage(i)=0;
            Volt_Flag=1;
            
        elseif voltages(i) < min_threshold
            Under_voltage(i)=0;
            Volt_Flag =1 ;
        end
        Switchs(i)=Under_voltage(i)||Over_voltage(i);
    end 
end

== stateflow chart Final_V2/The whole system/Master/Chart1 ==
  state Charge_State
    state Refrence_check
      entry:
      New_Refrence=min_cell;
      update_refrence=New_Refrence-Refrence
    state New_state_check
      entry:
      n=1
      during :
      for n = 1:14
      if (cell_Soc(n) > min_cell)
      update_flag(n) = 1;
      end
      end
      end
    state initialization_of_switches
      entry:
      balance_enable=zeros(1,14);
      update_flag =ones(1,14);
      counter = zeros(1, 14);
      Balancing_Led=0;
    state init
      entry:
      V_difference=0.5;
      Battery_cap=2.2;
      Charging_Current=2.6;
      Balancing_Led=1;
    state protection_counter
      entry:
      pack_switch = 1;
      k = 1;  % Initialize k
      during:
      for k = 1:14
      if cell_Soc(k) == min_cell
      counter(k) = counter(k) + 1;
      if counter(k) > 10
      pack_switch = 0;
      end
      else
      counter(k) = 0;
      end
      end
    state Discharge_Time_interval
      entry:
      G = 1;
      Time_delay = zeros(1, 14);
      during :
      for G = 1:14
      Discharge_current= 0.3;
      Time_delay(G) = ((2.2* (cell_Soc(G) - min_cell)) / Discharge_current)*60*60;
      end
      end
    state Data_Check
      i = 1;
      Refrence = min_cell;
      % Main loop
      for i = 1:14
      if update_flag(i) == 1
      if (cell_Soc(i) > Refrence && (cell_Soc(i) > 0.2))
      balance_enable(i) = 1;
      else
      balance_enable(i) = 0;
      end
      end
      end
    state Estimated_Time
      entry:
      Time_of_INC_V=0;
      Time_of_INC_V=(60*60*Battery_cap*V_difference)/Charging_Current;
    state Data_check [commented out]
      entry:
      i=1;
      Refrence=min_cell;
      during :
      for i = 1:14
      if (cell_Soc(i) > Refrence) && (cell_Soc(i) > 0.76)
      balance_enable(i) = 1;
      end
      end
      end
    Refrence_check -> Refrence_check [order 1] : after(1,sec)[update_refrence<0.01]
    New_state_check -> Charge_State.initialization_of_switches [order 1] : [(error==0) &&(n==14) ]
    Refrence_check -> New_state_check [order 2] : [update_refrence>0.02]
    Estimated_Time -> Refrence_check : after(Time_of_INC_V,sec)
    New_state_check -> Data_Check [order 2] : [(error==1) &&(n==14) ]
    (default) -> Charge_State.initialization_of_switches
    Charge_State.initialization_of_switches -> Data_Check : [error==1 && System_State==2]
    protection_counter -> init : [k==14]
    init -> Estimated_Time
    Charge_State.Discharge_Time_interval -> protection_counter : [G==14]
    Data_Check -> Charge_State.Discharge_Time_interval : [i==14]
  state Static_State
    state initialization_of_switches
      entry:
      balance_enable=zeros(1,14);
      Balancing_Led=0;
    state Counter_check
      entry:
      H= 1;
      min_time = -inf;
      during :
      if H <= 14
      if (Time_delay(H) > min_time) && (Time_delay(H) > 0)
      min_time = (Time_delay(H) * 60 * 60); % Update the maximum value
      end
      H = H + 1;
      end
    state Discharge_Time_interval
      entry:
      G = 1;
      Balancing_Led=1;
      Time_delay = zeros(1, 14);
      during :
      for G = 1:14
      Discharge_current= 0.5;
      Time_delay(G) = (2.2*60*60*(cell_Soc(G) - min_cell)) / Discharge_current;% cell capacity
      end
      end
    state Data_check
      entry:
      i=1;
      during :
      for i = 1:14
      if (cell_Soc(i) > min_cell) && (cell_Soc(i) > 0.2)
      balance_enable(i) = 1;
      end
      end
      end
    (default) -> Static_State.initialization_of_switches
    (junction 290) -> Static_State.Data_check [order 2] : [error==1]/{balance_enable=zeros(1,14)}
    (junction 290) -> Static_State.initialization_of_switches [order 1] : [error==0]{Balancing_Led=0}
    Counter_check -> (junction 290) : after(min_time,sec)[H==14]
    Static_State.initialization_of_switches -> Static_State.Data_check : [ error==1 && System_State==3 ]
    Static_State.Data_check -> Static_State.Discharge_Time_interval : [i==14]
    Static_State.Discharge_Time_interval -> Counter_check : [G==14]
  state Dis_Charging
    entry:
    balance_enable=zeros(1,14);
    update_flag =ones(1,14);
    counter = zeros(1, 14);
    Balancing_Led=0;
    Time_of_INC_V=0;
    min_time = 0;
    Time_delay = zeros(1, 14);
  (default) -> Static_State
  Charge_State -> Static_State [order 1] : [(System_State==3 )]
  Static_State -> Charge_State [order 1] : [(System_State==2)&& (error==1)]
  Dis_Charging -> Static_State [order 1] : [[(System_State==3)]]
  Dis_Charging -> Charge_State [order 2] : [[(System_State==2)]]
  Charge_State -> Dis_Charging [order 2] : [[(System_State==1)]]
  Static_State -> Dis_Charging [order 2] : [[(System_State==1)]]
  data: cell_Soc input double size=[1,14]; balance_enable output double size=[1,14]; i local; System_State input; V_difference local; Time_of_INC_V local; Battery_cap local; New_Refrence local; Refrence local; n local; update_flag local double size=[1,14]; Charging_Current local; G local; H local; k local; pack_switch output; Time_delay output; update_refrence local; Discharge_current local; min_time local; Balancing_Led output; counter local double size=[1,14]; error input; min_cell input
